simulink model "x_vacc_test"  (Simulink 8.0)
settings: Solver=ode45  StartTime=0.0  StopTime=20*(4096/8)*40  RelTol=1e-3
model workspace (MAT data in the model file):
  ws_data_in.time = [0 1 2 3 4 5 6 7 8 9 10 11 ... (163840 elements, 163840x1)]
  ws_data_in.signals.values = [1 0 0 0 0 0 0 0 0 0 0 0 ... (163840 elements, 163840x1)]
  ws_valid_in.time = [0 1 2 3 4 5 6 7 8 9 10 11 ... (163840 elements, 163840x1)]
  ws_valid_in.signals.values = [1 1 1 1 1 1 1 1 1 1 1 0 ... (163840 elements, 163840x1)]
  ws_dumps_per_acc_in.time = 0
  ws_dumps_per_acc_in.signals.values = 2
libraries: xbsIndex_r4 x531
(part 1 of 4)
settings Model: Creator=davidm  LastModifiedBy=davidm

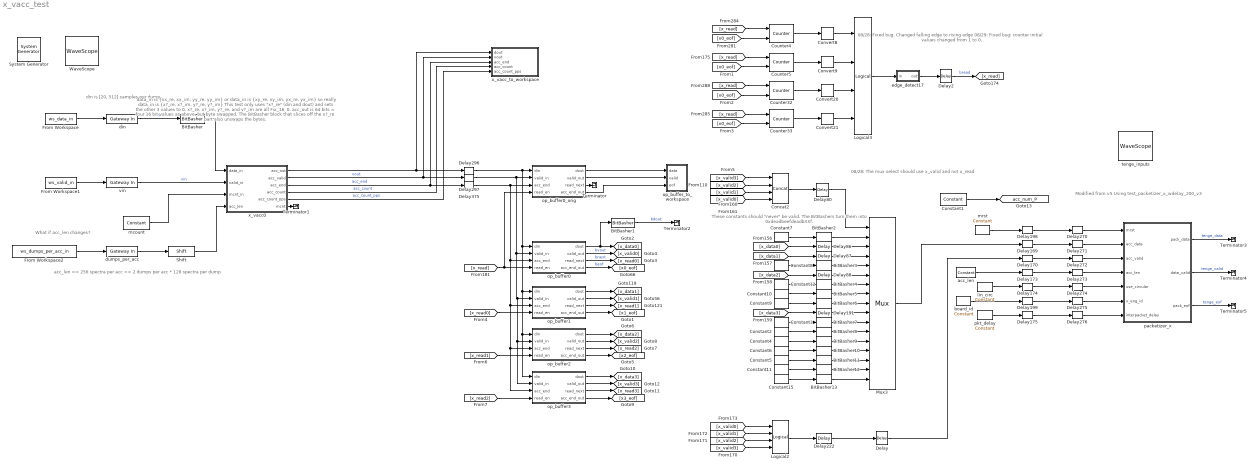
== system x_vacc_test ==
  note: What if acc_len changes?
  note: din is [20, 512] samples per dump
  note: acc_len == 256 spectra per acc == 2 dumps per acc * 128 spectra per dump
  note: data_in  is {xx_re, xx_im, yy_re, yy_im} or
      | data_in  is {xy_re, xy_im, yx_re, yx_im} so really
      | data_in is {x?_re, x?_im, y?_re, y?_im}
      | This test only uses "x?_re" (din and dout) and sets the other 3 values to 0.
      | x?_re, x?_im, y?_re, and y?_im are all Fix_16_0.
      |
      | acc_out is 64 bits = four 16 bit values as above, but byte swapped.
      | The BitBasher block that slices off the x?_re part also unswaps the bytes.
  note: 08/28: Fixed bug. Changed falling edge to rising edge
      | 08/29: Fixed bug: counter initial values changed from 1 to 0.
  note: These constants should "never" be valid.
      | The BitBashers turn them into
      | 0xdeadbeefdeadbXXf.
  note: Modified from v5
      | Using  test_packetizer_x_wdelay_200_v3
  note: 08/28: The mux select should use x_valid and not x_read
  " System Generator" : xbsIndex_r4/ System Generator  Tag=genX  xilinxfamily=Kintex7  part=xc7k325t  speed=-3  package=fbg676  synthesis_tool=XST  clock_wrapper=Clock Enables  directory=./netlist  proj_type=Project Navigator  Synth_file=XST Defaults  Impl_file=ISE Defaults  testbench=off  simulink_period=1  sysclk_period=10  dcm_input_clock_period=10  incr_netlist=off  trim_vbits=Everywhere in SubSystem  dbl_ovrd=According to Block Masks  core_generation=According to Block Masks  run_coregen=off  deprecated_control=off  eval_field=0  block_type=sysgen
  BitBasher : xbsIndex_r4/BitBasher  bitexpr=|  display_expr=off  sr_1=1  arith_type1=Unsigned  bin_pt1=0  sr_2=2  arith_type2=Unsigned  bin_pt2=0  sr_3=3  arith_type3=Unsigned  bin_pt3=0  sr_4=4  arith_type4=Unsigned  bin_pt4=0  dbl_ovrd=off  block_type=bitbasher
      | <!DOCTYPE HTML PUBLIC "-//W3C//DTD HTML 4.0//EN" "http://www.w3.org/TR/REC-html40/strict.dtd">
      | <html><head><meta name="qrichtext" content="1" /><style type="text/css">
      | p, li { white-space: pre-wrap; }
      | </style></head><body style=" font-family:'Sans'; font-size:10pt; font-weight:400; font-style:normal;">
      | <p style=" margin-top:12px; margin-bottom:12px; margin-left:0px; margin-right:0px; -qt-block-indent:0; text-indent:0px;"><span style=" font-family:'MS Shell Dlg'; font-size:8pt;">d64={d, 48'b0}</span></p></body></html>
  BitBasher1 : xbsIndex_r4/BitBasher  bitexpr=|  display_expr=off  sr_1=1  arith_type1="Signed  (2's comp)"  bin_pt1=0  sr_2=2  arith_type2=Unsigned  bin_pt2=0  sr_3=3  arith_type3=Unsigned  bin_pt3=0  sr_4=4  arith_type4=Unsigned  bin_pt4=0  dbl_ovrd=off  block_type=bitbasher
      | <!DOCTYPE HTML PUBLIC "-//W3C//DTD HTML 4.0//EN" "http://www.w3.org/TR/REC-html40/strict.dtd">
      | <html><head><meta name="qrichtext" content="1" /><style type="text/css">
      | p, li { white-space: pre-wrap; }
      | </style></head><body style=" font-family:'Sans'; font-size:10pt; font-weight:400; font-style:normal;">
      | <p style=" margin-top:12px; margin-bottom:12px; margin-left:0px; margin-right:0px; -qt-block-indent:0; text-indent:0px;"><span style=" font-family:'MS Shell Dlg'; font-size:8pt;">d16={d[55:48],d[63:56]}</span></p></body></html>
  BitBasher10 : xbsIndex_r4/BitBasher  bitexpr=|  display_expr=off  sr_1=1  arith_type1=Unsigned  bin_pt1=0  sr_2=2  arith_type2=Unsigned  bin_pt2=0  sr_3=3  arith_type3=Unsigned  bin_pt3=0  sr_4=4  arith_type4=Unsigned  bin_pt4=0  dbl_ovrd=off  block_type=bitbasher
      | <!DOCTYPE HTML PUBLIC "-//W3C//DTD HTML 4.0//EN" "http://www.w3.org/TR/REC-html40/strict.dtd">
      | <html><head><meta name="qrichtext" content="1" /><style type="text/css">
      | p, li { white-space: pre-wrap; }
      | </style></head><body style=" font-family:'Sans'; font-size:10pt; font-weight:400; font-style:normal;">
      | <p style=" margin-top:12px; margin-bottom:12px; margin-left:0px; margin-right:0px; -qt-block-indent:0; text-indent:0px;"><span style=" font-family:'MS Shell Dlg'; font-size:8pt;">o={32'hdeadbeef, 16'hdead, 4'hb, i[3:0], i[3:0], 4'hf}</span></p></body></html>
  BitBasher11 : xbsIndex_r4/BitBasher  bitexpr=|  display_expr=off  sr_1=1  arith_type1=Unsigned  bin_pt1=0  sr_2=2  arith_type2=Unsigned  bin_pt2=0  sr_3=3  arith_type3=Unsigned  bin_pt3=0  sr_4=4  arith_type4=Unsigned  bin_pt4=0  dbl_ovrd=off  block_type=bitbasher
      | <!DOCTYPE HTML PUBLIC "-//W3C//DTD HTML 4.0//EN" "http://www.w3.org/TR/REC-html40/strict.dtd">
      | <html><head><meta name="qrichtext" content="1" /><style type="text/css">
      | p, li { white-space: pre-wrap; }
      | </style></head><body style=" font-family:'Sans'; font-size:10pt; font-weight:400; font-style:normal;">
      | <p style=" margin-top:12px; margin-bottom:12px; margin-left:0px; margin-right:0px; -qt-block-indent:0; text-indent:0px;"><span style=" font-family:'MS Shell Dlg'; font-size:8pt;">o={32'hdeadbeef, 16'hdead, 4'hb, i[3:0], i[3:0], 4'hf}</span></p></body></html>
  BitBasher12 : xbsIndex_r4/BitBasher  bitexpr=|  display_expr=off  sr_1=1  arith_type1=Unsigned  bin_pt1=0  sr_2=2  arith_type2=Unsigned  bin_pt2=0  sr_3=3  arith_type3=Unsigned  bin_pt3=0  sr_4=4  arith_type4=Unsigned  bin_pt4=0  dbl_ovrd=off  block_type=bitbasher
      | <!DOCTYPE HTML PUBLIC "-//W3C//DTD HTML 4.0//EN" "http://www.w3.org/TR/REC-html40/strict.dtd">
      | <html><head><meta name="qrichtext" content="1" /><style type="text/css">
      | p, li { white-space: pre-wrap; }
      | </style></head><body style=" font-family:'Sans'; font-size:10pt; font-weight:400; font-style:normal;">
      | <p style=" margin-top:12px; margin-bottom:12px; margin-left:0px; margin-right:0px; -qt-block-indent:0; text-indent:0px;"><span style=" font-family:'MS Shell Dlg'; font-size:8pt;">o={32'hdeadbeef, 16'hdead, 4'hb, i[3:0], i[3:0], 4'hf}</span></p></body></html>
  BitBasher13 : xbsIndex_r4/BitBasher  bitexpr=|  display_expr=off  sr_1=1  arith_type1=Unsigned  bin_pt1=0  sr_2=2  arith_type2=Unsigned  bin_pt2=0  sr_3=3  arith_type3=Unsigned  bin_pt3=0  sr_4=4  arith_type4=Unsigned  bin_pt4=0  dbl_ovrd=off  block_type=bitbasher
      | <!DOCTYPE HTML PUBLIC "-//W3C//DTD HTML 4.0//EN" "http://www.w3.org/TR/REC-html40/strict.dtd">
      | <html><head><meta name="qrichtext" content="1" /><style type="text/css">
      | p, li { white-space: pre-wrap; }
      | </style></head><body style=" font-family:'Sans'; font-size:10pt; font-weight:400; font-style:normal;">
      | <p style=" margin-top:12px; margin-bottom:12px; margin-left:0px; margin-right:0px; -qt-block-indent:0; text-indent:0px;"><span style=" font-family:'MS Shell Dlg'; font-size:8pt;">o={32'hdeadbeef, 16'hdead, 4'hb, i[3:0], i[3:0], 4'hf}</span></p></body></html>
  BitBasher2 : xbsIndex_r4/BitBasher  bitexpr=|  display_expr=off  sr_1=1  arith_type1=Unsigned  bin_pt1=0  sr_2=2  arith_type2=Unsigned  bin_pt2=0  sr_3=3  arith_type3=Unsigned  bin_pt3=0  sr_4=4  arith_type4=Unsigned  bin_pt4=0  dbl_ovrd=off  block_type=bitbasher
      | <!DOCTYPE HTML PUBLIC "-//W3C//DTD HTML 4.0//EN" "http://www.w3.org/TR/REC-html40/strict.dtd">
      | <html><head><meta name="qrichtext" content="1" /><style type="text/css">
      | p, li { white-space: pre-wrap; }
      | </style></head><body style=" font-family:'Sans'; font-size:10pt; font-weight:400; font-style:normal;">
      | <p style=" margin-top:12px; margin-bottom:12px; margin-left:0px; margin-right:0px; -qt-block-indent:0; text-indent:0px;"><span style=" font-family:'MS Shell Dlg'; font-size:8pt;">o={32'hdeadbeef, 16'hdead, 4'hb, i[3:0], i[3:0], 4'hf}</span></p></body></html>
  BitBasher3 : xbsIndex_r4/BitBasher  bitexpr=|  display_expr=off  sr_1=1  arith_type1=Unsigned  bin_pt1=0  sr_2=2  arith_type2=Unsigned  bin_pt2=0  sr_3=3  arith_type3=Unsigned  bin_pt3=0  sr_4=4  arith_type4=Unsigned  bin_pt4=0  dbl_ovrd=off  block_type=bitbasher
      | <!DOCTYPE HTML PUBLIC "-//W3C//DTD HTML 4.0//EN" "http://www.w3.org/TR/REC-html40/strict.dtd">
      | <html><head><meta name="qrichtext" content="1" /><style type="text/css">
      | p, li { white-space: pre-wrap; }
      | </style></head><body style=" font-family:'Sans'; font-size:10pt; font-weight:400; font-style:normal;">
      | <p style=" margin-top:12px; margin-bottom:12px; margin-left:0px; margin-right:0px; -qt-block-indent:0; text-indent:0px;"><span style=" font-family:'MS Shell Dlg'; font-size:8pt;">o={32'hdeadbeef, 16'hdead, 4'hb, i[3:0], i[3:0], 4'hf}</span></p></body></html>
  BitBasher4 : xbsIndex_r4/BitBasher  bitexpr=|  display_expr=off  sr_1=1  arith_type1=Unsigned  bin_pt1=0  sr_2=2  arith_type2=Unsigned  bin_pt2=0  sr_3=3  arith_type3=Unsigned  bin_pt3=0  sr_4=4  arith_type4=Unsigned  bin_pt4=0  dbl_ovrd=off  block_type=bitbasher
      | <!DOCTYPE HTML PUBLIC "-//W3C//DTD HTML 4.0//EN" "http://www.w3.org/TR/REC-html40/strict.dtd">
      | <html><head><meta name="qrichtext" content="1" /><style type="text/css">
      | p, li { white-space: pre-wrap; }
      | </style></head><body style=" font-family:'Sans'; font-size:10pt; font-weight:400; font-style:normal;">
      | <p style=" margin-top:12px; margin-bottom:12px; margin-left:0px; margin-right:0px; -qt-block-indent:0; text-indent:0px;"><span style=" font-family:'MS Shell Dlg'; font-size:8pt;">o={32'hdeadbeef, 16'hdead, 4'hb, i[3:0], i[3:0], 4'hf}</span></p></body></html>
  BitBasher5 : xbsIndex_r4/BitBasher  bitexpr=|  display_expr=off  sr_1=1  arith_type1=Unsigned  bin_pt1=0  sr_2=2  arith_type2=Unsigned  bin_pt2=0  sr_3=3  arith_type3=Unsigned  bin_pt3=0  sr_4=4  arith_type4=Unsigned  bin_pt4=0  dbl_ovrd=off  block_type=bitbasher
      | <!DOCTYPE HTML PUBLIC "-//W3C//DTD HTML 4.0//EN" "http://www.w3.org/TR/REC-html40/strict.dtd">
      | <html><head><meta name="qrichtext" content="1" /><style type="text/css">
      | p, li { white-space: pre-wrap; }
      | </style></head><body style=" font-family:'Sans'; font-size:10pt; font-weight:400; font-style:normal;">
      | <p style=" margin-top:12px; margin-bottom:12px; margin-left:0px; margin-right:0px; -qt-block-indent:0; text-indent:0px;"><span style=" font-family:'MS Shell Dlg'; font-size:8pt;">o={32'hdeadbeef, 16'hdead, 4'hb, i[3:0], i[3:0], 4'hf}</span></p></body></html>
  BitBasher6 : xbsIndex_r4/BitBasher  bitexpr=|  display_expr=off  sr_1=1  arith_type1=Unsigned  bin_pt1=0  sr_2=2  arith_type2=Unsigned  bin_pt2=0  sr_3=3  arith_type3=Unsigned  bin_pt3=0  sr_4=4  arith_type4=Unsigned  bin_pt4=0  dbl_ovrd=off  block_type=bitbasher
      | <!DOCTYPE HTML PUBLIC "-//W3C//DTD HTML 4.0//EN" "http://www.w3.org/TR/REC-html40/strict.dtd">
      | <html><head><meta name="qrichtext" content="1" /><style type="text/css">
      | p, li { white-space: pre-wrap; }
      | </style></head><body style=" font-family:'Sans'; font-size:10pt; font-weight:400; font-style:normal;">
      | <p style=" margin-top:12px; margin-bottom:12px; margin-left:0px; margin-right:0px; -qt-block-indent:0; text-indent:0px;"><span style=" font-family:'MS Shell Dlg'; font-size:8pt;">o={32'hdeadbeef, 16'hdead, 4'hb, i[3:0], i[3:0], 4'hf}</span></p></body></html>
  BitBasher7 : xbsIndex_r4/BitBasher  bitexpr=|  display_expr=off  sr_1=1  arith_type1=Unsigned  bin_pt1=0  sr_2=2  arith_type2=Unsigned  bin_pt2=0  sr_3=3  arith_type3=Unsigned  bin_pt3=0  sr_4=4  arith_type4=Unsigned  bin_pt4=0  dbl_ovrd=off  block_type=bitbasher
      | <!DOCTYPE HTML PUBLIC "-//W3C//DTD HTML 4.0//EN" "http://www.w3.org/TR/REC-html40/strict.dtd">
      | <html><head><meta name="qrichtext" content="1" /><style type="text/css">
      | p, li { white-space: pre-wrap; }
      | </style></head><body style=" font-family:'Sans'; font-size:10pt; font-weight:400; font-style:normal;">
      | <p style=" margin-top:12px; margin-bottom:12px; margin-left:0px; margin-right:0px; -qt-block-indent:0; text-indent:0px;"><span style=" font-family:'MS Shell Dlg'; font-size:8pt;">o={32'hdeadbeef, 16'hdead, 4'hb, i[3:0], i[3:0], 4'hf}</span></p></body></html>
  BitBasher8 : xbsIndex_r4/BitBasher  bitexpr=|  display_expr=off  sr_1=1  arith_type1=Unsigned  bin_pt1=0  sr_2=2  arith_type2=Unsigned  bin_pt2=0  sr_3=3  arith_type3=Unsigned  bin_pt3=0  sr_4=4  arith_type4=Unsigned  bin_pt4=0  dbl_ovrd=off  block_type=bitbasher
      | <!DOCTYPE HTML PUBLIC "-//W3C//DTD HTML 4.0//EN" "http://www.w3.org/TR/REC-html40/strict.dtd">
      | <html><head><meta name="qrichtext" content="1" /><style type="text/css">
      | p, li { white-space: pre-wrap; }
      | </style></head><body style=" font-family:'Sans'; font-size:10pt; font-weight:400; font-style:normal;">
      | <p style=" margin-top:12px; margin-bottom:12px; margin-left:0px; margin-right:0px; -qt-block-indent:0; text-indent:0px;"><span style=" font-family:'MS Shell Dlg'; font-size:8pt;">o={32'hdeadbeef, 16'hdead, 4'hb, i[3:0], i[3:0], 4'hf}</span></p></body></html>
  BitBasher9 : xbsIndex_r4/BitBasher  bitexpr=|  display_expr=off  sr_1=1  arith_type1=Unsigned  bin_pt1=0  sr_2=2  arith_type2=Unsigned  bin_pt2=0  sr_3=3  arith_type3=Unsigned  bin_pt3=0  sr_4=4  arith_type4=Unsigned  bin_pt4=0  dbl_ovrd=off  block_type=bitbasher
      | <!DOCTYPE HTML PUBLIC "-//W3C//DTD HTML 4.0//EN" "http://www.w3.org/TR/REC-html40/strict.dtd">
      | <html><head><meta name="qrichtext" content="1" /><style type="text/css">
      | p, li { white-space: pre-wrap; }
      | </style></head><body style=" font-family:'Sans'; font-size:10pt; font-weight:400; font-style:normal;">
      | <p style=" margin-top:12px; margin-bottom:12px; margin-left:0px; margin-right:0px; -qt-block-indent:0; text-indent:0px;"><span style=" font-family:'MS Shell Dlg'; font-size:8pt;">o={32'hdeadbeef, 16'hdead, 4'hb, i[3:0], i[3:0], 4'hf}</span></p></body></html>
  Concat2 : xbsIndex_r4/Concat  num_inputs=4  dbl_ovrd=off  block_type=concat
      settings: block_version=10.1.3
  Constant1 : xbsIndex_r4/Constant  const=0  gui_display_data_type=Fixed-point  arith_type=Unsigned  n_bits=32  bin_pt=0  preci_type=Single  exp_width=8  frac_width=24  explicit_period=on  period=1  equ=P=C  opselect=C  inp2=PCIN>>17  opr=+  inp1=P  carry=CIN  dbl_ovrd=off  block_type=constant
      settings: block_version=11.4
  Constant10 : xbsIndex_r4/Constant  const=6  gui_display_data_type=Fixed-point  arith_type=Unsigned  n_bits=4  bin_pt=0  preci_type=Single  exp_width=8  frac_width=24  explicit_period=on  period=1  equ=P=C  opselect=C  inp2=PCIN>>17  opr=+  inp1=P  carry=CIN  dbl_ovrd=off  block_type=constant
      settings: block_version=11.4
  Constant11 : xbsIndex_r4/Constant  const=14  gui_display_data_type=Fixed-point  arith_type=Unsigned  n_bits=4  bin_pt=0  preci_type=Single  exp_width=8  frac_width=24  explicit_period=on  period=1  equ=P=C  opselect=C  inp2=PCIN>>17  opr=+  inp1=P  carry=CIN  dbl_ovrd=off  block_type=constant
      settings: block_version=11.4
  Constant12 : xbsIndex_r4/Constant  const=5  gui_display_data_type=Fixed-point  arith_type=Unsigned  n_bits=4  bin_pt=0  preci_type=Single  exp_width=8  frac_width=24  explicit_period=on  period=1  equ=P=C  opselect=C  inp2=PCIN>>17  opr=+  inp1=P  carry=CIN  dbl_ovrd=off  block_type=constant
      settings: block_version=11.4
  Constant15 : xbsIndex_r4/Constant  const=15  gui_display_data_type=Fixed-point  arith_type=Unsigned  n_bits=4  bin_pt=0  preci_type=Single  exp_width=8  frac_width=24  explicit_period=on  period=1  equ=P=C  opselect=C  inp2=PCIN>>17  opr=+  inp1=P  carry=CIN  dbl_ovrd=off  block_type=constant
      settings: block_version=11.4
  Constant2 : xbsIndex_r4/Constant  const=10  gui_display_data_type=Fixed-point  arith_type=Unsigned  n_bits=4  bin_pt=0  preci_type=Single  exp_width=8  frac_width=24  explicit_period=on  period=1  equ=P=C  opselect=C  inp2=PCIN>>17  opr=+  inp1=P  carry=CIN  dbl_ovrd=off  block_type=constant
      settings: block_version=11.4
  Constant3 : xbsIndex_r4/Constant  const=9  gui_display_data_type=Fixed-point  arith_type=Unsigned  n_bits=4  bin_pt=0  preci_type=Single  exp_width=8  frac_width=24  explicit_period=on  period=1  equ=P=C  opselect=C  inp2=PCIN>>17  opr=+  inp1=P  carry=CIN  dbl_ovrd=off  block_type=constant
      settings: block_version=11.4
  Constant4 : xbsIndex_r4/Constant  const=11  gui_display_data_type=Fixed-point  arith_type=Unsigned  n_bits=4  bin_pt=0  preci_type=Single  exp_width=8  frac_width=24  explicit_period=on  period=1  equ=P=C  opselect=C  inp2=PCIN>>17  opr=+  inp1=P  carry=CIN  dbl_ovrd=off  block_type=constant
      settings: block_version=11.4
  Constant5 : xbsIndex_r4/Constant  const=13  gui_display_data_type=Fixed-point  arith_type=Unsigned  n_bits=4  bin_pt=0  preci_type=Single  exp_width=8  frac_width=24  explicit_period=on  period=1  equ=P=C  opselect=C  inp2=PCIN>>17  opr=+  inp1=P  carry=CIN  dbl_ovrd=off  block_type=constant
      settings: block_version=11.4
  Constant6 : xbsIndex_r4/Constant  const=12  gui_display_data_type=Fixed-point  arith_type=Unsigned  n_bits=4  bin_pt=0  preci_type=Single  exp_width=8  frac_width=24  explicit_period=on  period=1  equ=P=C  opselect=C  inp2=PCIN>>17  opr=+  inp1=P  carry=CIN  dbl_ovrd=off  block_type=constant
      settings: block_version=11.4
  Constant7 : xbsIndex_r4/Constant  const=0  gui_display_data_type=Fixed-point  arith_type=Unsigned  n_bits=4  bin_pt=0  preci_type=Single  exp_width=8  frac_width=24  explicit_period=on  period=1  equ=P=C  opselect=C  inp2=PCIN>>17  opr=+  inp1=P  carry=CIN  dbl_ovrd=off  block_type=constant
      settings: block_version=11.4
  Constant8 : xbsIndex_r4/Constant  const=3  gui_display_data_type=Fixed-point  arith_type=Unsigned  n_bits=4  bin_pt=0  preci_type=Single  exp_width=8  frac_width=24  explicit_period=on  period=1  equ=P=C  opselect=C  inp2=PCIN>>17  opr=+  inp1=P  carry=CIN  dbl_ovrd=off  block_type=constant
      settings: block_version=11.4
  Constant9 : xbsIndex_r4/Constant  const=7  gui_display_data_type=Fixed-point  arith_type=Unsigned  n_bits=4  bin_pt=0  preci_type=Single  exp_width=8  frac_width=24  explicit_period=on  period=1  equ=P=C  opselect=C  inp2=PCIN>>17  opr=+  inp1=P  carry=CIN  dbl_ovrd=off  block_type=constant
      settings: block_version=11.4
  Convert{8..9,20..21} (4 alike) : xbsIndex_r4/Convert  gui_display_data_type=Fixed-point  arith_type=Boolean  n_bits=16  bin_pt=14  float_type=Single  exp_bits=8  fraction_bits=24  quantization=Truncate  overflow=Wrap  en=off  latency=0  dbl_ovrd=off  pipeline=on  block_type=convert
      settings: block_version=11.4
  Counter{4..5,32..33} (4 alike) : xbsIndex_r4/Counter  cnt_type=Free Running  cnt_to=Inf  operation=Up  start_count=0  cnt_by_val=1  arith_type=Unsigned  n_bits=1  bin_pt=0  load_pin=off  rst=on  en=on  explicit_period=off  period=1  dbl_ovrd=off  use_behavioral_HDL=off  implementation=Fabric  block_type=counter
      settings: block_version=11.4
  Delay{,2,80,86..88,191,222,270..276,296..297,375} (18 alike) : xbsIndex_r4/Delay  rst=off  infoeditControl=Selection of Reset will increase slice count due to use of real FFs and instead of SRLs  en=off  latency=1  dbl_ovrd=off  reg_retiming=on  block_type=delay
      settings: block_version=11.4
  Delay{169..170,173..175,198..199} (7 alike) : xbsIndex_r4/Delay  rst=off  infoeditControl=Selection of Reset will increase slice count due to use of real FFs and instead of SRLs  en=off  latency=2  dbl_ovrd=off  reg_retiming=on  block_type=delay
      settings: block_version=11.4
  From Workspace : FromWorkspace  VariableName=ws_data_in  SampleTime=1  Interpolate=off  OutputAfterFinalValue=Holding final value
  From Workspace1 : FromWorkspace  VariableName=ws_valid_in  SampleTime=1  Interpolate=off  OutputAfterFinalValue=Holding final value
  From Workspace2 : FromWorkspace  VariableName=ws_dumps_per_acc_in  SampleTime=1  Interpolate=off  OutputAfterFinalValue=Holding final value
  Logical2 : xbsIndex_r4/Logical  logical_function=OR  inputs=4  en=off  latency=0  precision=Full  arith_type=Unsigned  n_bits=16  bin_pt=0  align_bp=on  dbl_ovrd=off  block_type=logical
      settings: block_version=11.4
  Logical3 : xbsIndex_r4/Logical  logical_function=AND  inputs=4  en=off  latency=0  precision=Full  arith_type=Unsigned  n_bits=16  bin_pt=0  align_bp=on  dbl_ovrd=off  block_type=logical
      settings: block_version=11.4
  Mux3 : xbsIndex_r4/Mux  inputs=16  en=off  latency=1  precision=Full  arith_type=Unsigned  n_bits=16  bin_pt=14  quantization=Truncate  overflow=Wrap  dbl_ovrd=off  block_type=mux
      settings: block_version=11.4
  Shift : xbsIndex_r4/Shift  shift_dir=Left  shift_bits=7  en=off  latency=0  precision=Full  arith_type="Signed  (2's comp)"  n_bits=16  bin_pt=14  quantization=Truncate  overflow=Wrap  dbl_ovrd=off  block_type=shift
  Terminator{,1..5} (6 alike) : Terminator
  WaveScope : xbsIndex_r4/WaveScope  block_type=wavescope
  acc_len : xbsIndex_r4/Constant  const=2  gui_display_data_type=Fixed-point  arith_type=Unsigned  n_bits=16  bin_pt=0  preci_type=Single  exp_width=8  frac_width=24  explicit_period=on  period=1  equ=P=C  opselect=C  inp2=PCIN>>17  opr=+  inp1=P  carry=CIN  dbl_ovrd=off  block_type=constant
      settings: block_version=11.4
  board_id : xbsIndex_r4/Constant  const=0  gui_display_data_type=Fixed-point  arith_type=Unsigned  n_bits=4  bin_pt=0  preci_type=Single  exp_width=8  frac_width=24  explicit_period=on  period=1  equ=P=C  opselect=C  inp2=PCIN>>17  opr=+  inp1=P  carry=CIN  dbl_ovrd=off  block_type=constant
      settings: block_version=11.4
  din : xbsIndex_r4/Gateway In  gui_display_data_type=Fixed-point  arith_type="Signed  (2's comp)"  n_bits=16  bin_pt=0  preci_type=Single  exp_width=8  frac_width=24  quantization="Round  (unbiased: +/- Inf)"  overflow=Saturate  period=1  dbl_ovrd=off  timing_constraint=None  locs_specified=off  LOCs={}  UseAsADC=off  ADCChannel='1'  inherit_from_input=off  hdl_port=on  block_type=gatewayin
  dumps_per_acc : xbsIndex_r4/Gateway In  gui_display_data_type=Fixed-point  arith_type=Unsigned  n_bits=16  bin_pt=0  preci_type=Single  exp_width=8  frac_width=24  quantization="Round  (unbiased: +/- Inf)"  overflow=Saturate  period=1  dbl_ovrd=off  timing_constraint=None  locs_specified=off  LOCs={}  UseAsADC=off  ADCChannel='1'  inherit_from_input=off  hdl_port=on  block_type=gatewayin
  edge_detect17 : Subsystem  AncestorBlock=casper_library_misc/edge_detect  mask(edge_detect){edge=Rising, polarity=Active High, x_in=[0 0.08 0.08 0.16 0.16 0.24 0.24 0.32 0.32 0.4], y_in=[-1 -1  1  1  1  1  1  1  1  1], x_out=[0.6 0.68 0.68 0.76 0.76 0.84 0.84 0.92 0.92 1], y_out=[-1 -1  1  1 -1 -1 -1 -1 -1 -1]}
      mask prompts: edge "Edge Type"; polarity "Output Polarity"; x_in "Input X Positions"; y_in "Input Y Positions"; x_out "Output X Positions"; y_out "Output Y Positions"
      mask help: Output is active for one-cycle after each edge of the specified type (i.e. rising, falling or both).
      mask init:
      | edge_detect_init(gcb,...
      |     'edge',edge,...
      |     'polarity',polarity,...
      |     'x_in',x_in,...
      |     'x_out',x_out,...
      |     'y_in',y_in,...
      |     'y_out',y_out);
      | 
      | x_in_num=str2num(getfield(getfield(get_param(gcb,'UserData'),'parameters'),'x_in'));
      | x_out_num=str2num(getfield(getfield(get_param(gcb,'UserData'),'parameters'),'x_out'));
      | y_in_num=str2num(getfield(getfield(get_param(gcb,'UserData'),'parameters'),'y_in'));
      | y_out_num=str2num(getfield(getfield(get_param(gcb,'UserData'),'parameters'),'y_out'));
      settings: AttributesFormatString=|
      | rising edges
      | active high
      mask Help: Output is active for one-cycle after each edge of the specified type (i.e. rising, falling, or both).  Latency is zero.
      mask TypeOptions edge: Rising | Falling | Both
      mask TypeOptions polarity: Active High | Active Low
      mask Display:
      | plot(x_in_num,0.5+y_in_num*0.2);
      | plot(x_out_num,0.5+y_out_num*0.2);
  lin_circ : xbsIndex_r4/Constant  const=0  gui_display_data_type=Fixed-point  arith_type=Unsigned  n_bits=1  bin_pt=0  preci_type=Single  exp_width=8  frac_width=24  explicit_period=on  period=1  equ=P=C  opselect=C  inp2=PCIN>>17  opr=+  inp1=P  carry=CIN  dbl_ovrd=off  block_type=constant
      settings: block_version=11.4
  mcount : xbsIndex_r4/Constant  const=1  gui_display_data_type=Fixed-point  arith_type=Unsigned  n_bits=48  bin_pt=0  preci_type=Single  exp_width=8  frac_width=24  explicit_period=on  period=1  equ=P=C  opselect=C  inp2=PCIN>>17  opr=+  inp1=P  carry=CIN  dbl_ovrd=off  block_type=constant
  mrst : xbsIndex_r4/Constant  const=0  gui_display_data_type=Boolean  arith_type=Boolean  n_bits=4  bin_pt=0  preci_type=Single  exp_width=8  frac_width=24  explicit_period=on  period=1  equ=P=C  opselect=C  inp2=PCIN>>17  opr=+  inp1=P  carry=CIN  dbl_ovrd=off  block_type=constant
      settings: block_version=11.4
  op_buffer0 : Subsystem  mask{freq_chan=12, n_x_eng=3, n_x_op=22, n_buffer=1, bram_latency=2}
      mask prompts: freq_chan "No. of Frequency Channels in the system (2 ^ ?)"; n_x_eng "No. of X-engines in the system (2 ^ ?)"; n_x_op "No. of X-engine outputs"; n_buffer "No. of accumulations to buffer"; bram_latency "BRAM latency"
      mask init:
      | req_depth = (n_x_op/2) * (2 ^ (freq_chan - n_x_eng));
      | fifo_depth = 2^nextpow2(req_depth);
      | if fifo_depth < 1024
      |   set_param([gcb,'/FIFO'], 'depth', sprintf('%d', fifo_depth));
      | else
      |   set_param([gcb,'/FIFO'], 'depth', sprintf('%dK', fifo_depth/1024));
      | end
  op_buffer0_orig : Subsystem  mask{freq_chan=12, n_x_eng=3, n_x_op=22, n_buffer=1, bram_latency=2}
      mask prompts: freq_chan "No. of Frequency Channels in the system (2 ^ ?)"; n_x_eng "No. of X-engines in the system (2 ^ ?)"; n_x_op "No. of X-engine outputs"; n_buffer "No. of accumulations to buffer"; bram_latency "BRAM latency"
  op_buffer1 : Subsystem  mask{freq_chan=12, n_x_eng=3, n_x_op=22, n_buffer=1, bram_latency=2}
      mask prompts: freq_chan "No. of Frequency Channels in the system (2 ^ ?)"; n_x_eng "No. of X-engines in the system (2 ^ ?)"; n_x_op "No. of X-engine outputs"; n_buffer "No. of accumulations to buffer"; bram_latency "BRAM latency"
      mask init: same as x_vacc_test/op_buffer0
  op_buffer2 : Subsystem  mask{freq_chan=12, n_x_eng=3, n_x_op=22, n_buffer=1, bram_latency=2}
      mask prompts: freq_chan "No. of Frequency Channels in the system (2 ^ ?)"; n_x_eng "No. of X-engines in the system (2 ^ ?)"; n_x_op "No. of X-engine outputs"; n_buffer "No. of accumulations to buffer"; bram_latency "BRAM latency"
      mask init: same as x_vacc_test/op_buffer0
  op_buffer3 : Subsystem  mask{freq_chan=12, n_x_eng=3, n_x_op=22, n_buffer=1, bram_latency=2}
      mask prompts: freq_chan "No. of Frequency Channels in the system (2 ^ ?)"; n_x_eng "No. of X-engines in the system (2 ^ ?)"; n_x_op "No. of X-engine outputs"; n_buffer "No. of accumulations to buffer"; bram_latency "BRAM latency"
      mask init: same as x_vacc_test/op_buffer0
  op_buffer_to_workspace : Subsystem
  packetizer_x : Subsystem  mask{header_len=88, n_packets=128, freq_chan=12, n_x_op=22, n_x=1}
      mask prompts: header_len "Packet header length (bytes)"; n_packets "No. of packets per accumulation"; freq_chan "Total frequency channels ( 2 ^ ? )"; n_x_op "Number of X-engine Output"; n_x "Number of ROACH boards (2 ^ ?)"
      mask init:
      | n_freq_chan = 2 ^ (freq_chan - n_x);
      | data_len = n_x_op * n_freq_chan * 4 / n_packets;
  pkt_delay : xbsIndex_r4/Constant  const=100  gui_display_data_type=Fixed-point  arith_type=Unsigned  n_bits=8  bin_pt=0  preci_type=Single  exp_width=8  frac_width=24  explicit_period=on  period=1  equ=P=C  opselect=C  inp2=PCIN>>17  opr=+  inp1=P  carry=CIN  dbl_ovrd=off  block_type=constant
      settings: block_version=11.4
  tenge_inputs : xbsIndex_r4/WaveScope  block_type=wavescope
  vin : xbsIndex_r4/Gateway In  gui_display_data_type=Boolean  arith_type=Boolean  n_bits=16  bin_pt=14  preci_type=Single  exp_width=8  frac_width=24  quantization="Round  (unbiased: +/- Inf)"  overflow=Saturate  period=1  dbl_ovrd=off  timing_constraint=None  locs_specified=off  LOCs={}  UseAsADC=off  ADCChannel='1'  inherit_from_input=off  hdl_port=on  block_type=gatewayin
  x_vacc0 : Subsystem  mask{x_acc_bits=7, x_bin_pt=6, freq_chan=12, n_x_eng=3, x_n_op=22, bram_latency=3}
      mask prompts: x_acc_bits "X-engine Accumulation (2 ^ ?)"; x_bin_pt "X-engine Output Binary point"; freq_chan "Frequency channels in the system (2 ^ ?)"; n_x_eng "Total no. of X-engines in the system (2 ^ ?)"; x_n_op "No. of X outputs"; bram_latency "BRAM Latency"
      mask init:
      | x_freq_chan = freq_chan - n_x_eng;
      | x_len = x_n_op / 2 * (2 ^ x_freq_chan);
  x_vacc_to_workspace : Subsystem
  tag scope: acc_num_P=global
  wires:
  mcount/1 -> x_vacc0/3(mcnt_in)
  Shift/1 -> x_vacc0/4(acc_len)
  vin/1 -> x_vacc0/2(valid_in)  "vin"
  din/1 -> BitBasher/1  "din"
  BitBasher/1 -> x_vacc0/1(data_in)
  From Workspace/1 -> din/1
  From Workspace1/1 -> vin/1
  From Workspace2/1 -> dumps_per_acc/1
  dumps_per_acc/1 -> Shift/1
  x_vacc0/2(acc_valid) -> Delay297/1, x_vacc_to_workspace/2(vout)  "vout"
  x_vacc0/3(acc_end) -> Delay375/1, x_vacc_to_workspace/3(acc_end)  "acc_end"
  x_vacc0/4(acc_count) -> x_vacc_to_workspace/4(acc_count)  "acc_count"
  x_vacc0/5(acc_count_pps) -> x_vacc_to_workspace/5(acc_count_pps)  "acc_count_pps"
  op_buffer0_orig/2(valid_out) -> op_buffer_to_workspace/2(valid)
  op_buffer0_orig/1(dout) -> op_buffer_to_workspace/1(data)
  x_vacc0/1(acc_out) -> Delay296/1, x_vacc_to_workspace/1(dout)
  [x_read] -> op_buffer0_orig/4(read_en), op_buffer0/4(read_en)
  Delay296/1 -> op_buffer0_orig/1(din), op_buffer3/1(din), op_buffer2/1(din), op_buffer1/1(din), op_buffer0/1(din)
  Delay375/1 -> op_buffer0_orig/3(acc_end), op_buffer3/3(acc_end), op_buffer2/3(acc_end), op_buffer1/3(acc_end), op_buffer0/3(acc_end)
  op_buffer0_orig/4(acc_end_out) -> op_buffer_to_workspace/3(eof)
  edge_detect17/1(out) -> Delay2/1
  [x0_eof] -> Counter4/2
  [x0_eof] -> Counter5/2
  Counter4/1 -> Convert8/1
  Counter5/1 -> Convert9/1
  Logical3/1 -> edge_detect17/1(in)
  [x_read] -> Counter5/1
  [x_read] -> Counter4/1
  Delay2/1 -> [x_read]  "bread"
  Convert8/1 -> Logical3/1
  Convert9/1 -> Logical3/2
  [x0_eof] -> Counter32/2
  Counter32/1 -> Convert20/1
  Counter33/1 -> Convert21/1
  [x_read] -> Counter33/1
  [x_read] -> Counter32/1
  Convert20/1 -> Logical3/3
  Convert21/1 -> Logical3/4
  [x0_eof] -> Counter33/2
  op_buffer0_orig/3(read_next) -> Terminator/1
  x_vacc0/6(mcnt) -> Terminator1/1
  op_buffer0/1(dout) -> [x_data0], BitBasher1/1
  op_buffer0/2(valid_out) -> [x_valid0]  "bvout"
  op_buffer0/3(read_next) -> [x_read0]  "bnext"
  op_buffer0/4(acc_end_out) -> [x0_eof]  "beof"
  BitBasher1/1 -> Terminator2/1  "bdout"
  acc_len/1 -> Delay173/1
  lin_circ/1 -> Delay174/1
  board_id/1 -> Delay199/1
  mrst/1 -> Delay198/1
  Delay198/1 -> Delay270/1
  Delay169/1 -> Delay271/1
  Delay170/1 -> Delay272/1
  Delay173/1 -> Delay273/1
  Delay174/1 -> Delay274/1
  Delay199/1 -> Delay275/1
  pkt_delay/1 -> Delay175/1
  Delay175/1 -> Delay276/1
  Delay/1 -> Delay170/1
  Logical2/1 -> Delay222/1
  [x_data0] -> Delay86/1
  [x_valid0] -> Logical2/1
  [x_valid1] -> Logical2/2
  [x_valid2] -> Logical2/3
  [x_valid3] -> Logical2/4
  Mux3/1 -> Delay169/1
  Delay270/1 -> packetizer_x/1(mrst)
  Delay271/1 -> packetizer_x/2(acc_data)
  Delay272/1 -> packetizer_x/3(acc_valid)
  Delay273/1 -> packetizer_x/4(acc_len)
  Delay274/1 -> packetizer_x/5(use_circular)
  Delay275/1 -> packetizer_x/6(x_eng_id)
  Delay276/1 -> packetizer_x/7(interpacket_delay)
  Constant7/1 -> BitBasher2/1
  Delay86/1 -> Mux3/3
  [x_data1] -> Delay87/1
  [x_data2] -> Delay88/1
  [x_data3] -> Delay191/1
  Delay87/1 -> Mux3/4
  Constant8/1 -> BitBasher3/1
  Delay88/1 -> Mux3/6
  Delay191/1 -> Mux3/10
  Delay80/1 -> Mux3/1
  Delay222/1 -> Delay/1
  Concat2/1 -> Delay80/1
  [x_valid3] -> Concat2/1
  [x_valid2] -> Concat2/2
  [x_valid1] -> Concat2/3
  [x_valid0] -> Concat2/4
  BitBasher2/1 -> Mux3/2
  BitBasher3/1 -> Mux3/5
  Constant12/1 -> BitBasher4/1
  Constant10/1 -> BitBasher5/1
  Constant9/1 -> BitBasher6/1
  [x_read0] -> op_buffer1/4(read_en)
  op_buffer1/4(acc_end_out) -> [x1_eof]
  op_buffer1/1(dout) -> [x_data1]
  op_buffer1/2(valid_out) -> [x_valid1]
  op_buffer1/3(read_next) -> [x_read1]
  [x_read1] -> op_buffer2/4(read_en)
  op_buffer2/4(acc_end_out) -> [x2_eof]
  op_buffer2/1(dout) -> [x_data2]
  op_buffer2/2(valid_out) -> [x_valid2]
  op_buffer2/3(read_next) -> [x_read2]
  [x_read2] -> op_buffer3/4(read_en)
  op_buffer3/4(acc_end_out) -> [x3_eof]
  op_buffer3/1(dout) -> [x_data3]
  op_buffer3/2(valid_out) -> [x_valid3]
  op_buffer3/3(read_next) -> [x_read3]
  Delay297/1 -> op_buffer3/2(valid_in), op_buffer2/2(valid_in), op_buffer1/2(valid_in), op_buffer0/2(valid_in), op_buffer0_orig/2(valid_in)
  packetizer_x/1(pack_data) -> Terminator3/1  "tenge_data"
  packetizer_x/2(data_valid) -> Terminator4/1  "tenge_valid"
  packetizer_x/3(pack_eof) -> Terminator5/1  "tenge_eof"
  Constant1/1 -> [acc_num_P]
  BitBasher4/1 -> Mux3/7
  BitBasher5/1 -> Mux3/8
  BitBasher6/1 -> Mux3/9
  Constant3/1 -> BitBasher7/1
  Constant2/1 -> BitBasher8/1
  Constant4/1 -> BitBasher9/1
  BitBasher7/1 -> Mux3/11
  BitBasher8/1 -> Mux3/12
  BitBasher9/1 -> Mux3/13
  Constant6/1 -> BitBasher10/1
  Constant5/1 -> BitBasher11/1
  Constant11/1 -> BitBasher12/1
  BitBasher10/1 -> Mux3/14
  BitBasher11/1 -> Mux3/15
  BitBasher12/1 -> Mux3/16
  Constant15/1 -> BitBasher13/1
  BitBasher13/1 -> Mux3/17

== system x_vacc_test/edge_detect17 ==
  in : Inport
  Delay : xbsIndex_r4/Delay  rst=off  infoeditControl=Selection of Reset will increase slice count due to use of real FFs and instead of SRLs  en=off  latency=1  dbl_ovrd=off  reg_retiming=on  block_type=delay
      settings: xl_area=[0, 0, 0, 0, 0, 0, 0]
  Inverter : xbsIndex_r4/Inverter  en=off  latency=0  dbl_ovrd=off  block_type=inv
      settings: xl_area=[0, 0, 0, 0, 0, 0, 0]
  edge_op : xbsIndex_r4/Logical  logical_function=NOR  inputs=2  en=off  latency=0  precision=Full  arith_type=Unsigned  n_bits=8  bin_pt=2  align_bp=on  dbl_ovrd=off  block_type=logical
      settings: xl_area=[0, 0, 0, 0, 0, 0, 0]
  out : Outport
  wires:
  Inverter/1 -> edge_op/1
  edge_op/1 -> out/1
  in/1 -> Inverter/1, Delay/1
  Delay/1 -> edge_op/2

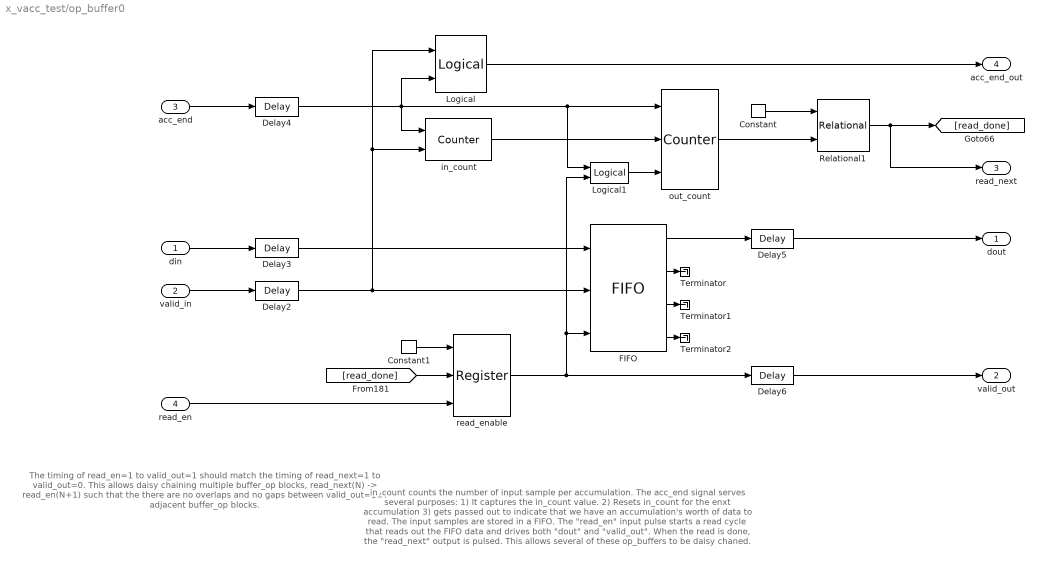
== system x_vacc_test/op_buffer0 ==
  note: The timing of read_en=1 to valid_out=1 should
      | match the timing of read_next=1 to valid_out=0.
      |
      | This allows daisy chaining multiple buffer_op blocks,
      | read_next(N) -> read_en(N+1) such that the there are
      | no overlaps and no gaps between valid_out=1 of
      | adjacent buffer_op blocks.
  note: in_count counts the number of input sample per accumulation.
      |
      | The acc_end signal serves several purposes:
      |
      | 1) It captures the in_count value.
      | 2) Resets in_count for the enxt accumulation
      | 3) gets passed out to indicate that we have an accumulation's
      | worth of data  to read.
      |
      | The input samples are stored in a FIFO.  The "read_en" input pulse
      | starts a read cycle that reads out the FIFO data and drives both
      | "dout" and "valid_out".  When the read is done, the "read_next"
      | output is pulsed.  This allows several of these op_buffers to be
      | daisy chaned.
  din : Inport
  valid_in : Inport  Port=2
  acc_end : Inport  Port=3
  read_en : Inport  Port=4
  Constant : xbsIndex_r4/Constant  const=1  gui_display_data_type=Fixed-point  arith_type=Unsigned  n_bits=1  bin_pt=0  preci_type=Single  exp_width=8  frac_width=24  explicit_period=on  period=1  equ=P=C  opselect=C  inp2=PCIN>>17  opr=+  inp1=P  carry=CIN  dbl_ovrd=off  block_type=constant
  Constant1 : xbsIndex_r4/Constant  const=1  gui_display_data_type=Boolean  arith_type=Boolean  n_bits=16  bin_pt=14  preci_type=Single  exp_width=8  frac_width=24  explicit_period=on  period=1  equ=P=C  opselect=C  inp2=PCIN>>17  opr=+  inp1=P  carry=CIN  dbl_ovrd=off  block_type=constant
  Delay{2..6} (5 alike) : xbsIndex_r4/Delay  rst=off  infoeditControl=Selection of Reset will increase slice count due to use of real FFs and instead of SRLs  en=off  latency=1  dbl_ovrd=off  reg_retiming=on  block_type=delay
      settings: block_version=11.4
  FIFO : xbsIndex_r4/FIFO  mem_type=Block RAM  performance_options=First_Word_Fall_Through  infoedit1=Available only for V4, V5, V6, V7, K7, A7 and Zynq devices.  embedded_registers=on  depth=8K  percent_nbits=1  rst=off  en=off  use_dcount=off  use_percent_full_port=on  use_almost_empty=off  almost_empty_thresh=2  use_almost_full=off  almost_full_thresh=14  dbl_ovrd=off  explicit_period=off  block_type=fifo
  Logical : xbsIndex_r4/Logical  logical_function=AND  inputs=2  en=off  latency=1  precision=Full  arith_type=Unsigned  n_bits=16  bin_pt=0  align_bp=on  dbl_ovrd=off  block_type=logical
  Logical1 : xbsIndex_r4/Logical  logical_function=OR  inputs=2  en=off  latency=0  precision=Full  arith_type=Unsigned  n_bits=16  bin_pt=0  align_bp=on  dbl_ovrd=off  block_type=logical
  Relational1 : xbsIndex_r4/Relational  mode=a=b  en=off  latency=1  dbl_ovrd=off  block_type=relational
  Terminator : Terminator
  Terminator1 : Terminator
  Terminator2 : Terminator
  in_count : xbsIndex_r4/Counter  cnt_type=Free Running  cnt_to=n_buffer * n_x_op * (2 ^ (freq_chan - n_x_eng)) - 1  operation=Up  start_count=0  cnt_by_val=1  arith_type=Unsigned  n_bits=nextpow2((n_x_op/2) * (2 ^ (freq_chan - n_x_eng)))  bin_pt=0  load_pin=off  rst=on  en=on  explicit_period=off  period=1  dbl_ovrd=off  use_behavioral_HDL=off  implementation=Fabric  block_type=counter
      settings: block_version=11.4
  out_count : xbsIndex_r4/Counter  cnt_type=Free Running  cnt_to=n_buffer * n_x_op * (2 ^ (freq_chan - n_x_eng)) - 1  operation=Down  start_count=0  cnt_by_val=1  arith_type=Unsigned  n_bits=nextpow2((n_x_op/2) * (2 ^ (freq_chan - n_x_eng)))  bin_pt=0  load_pin=on  rst=off  en=on  explicit_period=off  period=1  dbl_ovrd=off  use_behavioral_HDL=off  implementation=Fabric  block_type=counter
      settings: block_version=11.4
  read_enable : xbsIndex_r4/Register  init=0  rst=on  en=on  dbl_ovrd=off  block_type=register
  dout : Outport
  valid_out : Outport  Port=2
  read_next : Outport  Port=3
  acc_end_out : Outport  Port=4
  wires:
  acc_end/1 -> Delay4/1
  in_count/1 -> out_count/2
  valid_in/1 -> Delay2/1
  read_enable/1 -> Delay6/1, Logical1/2, FIFO/3
  out_count/1 -> Relational1/2
  din/1 -> Delay3/1
  Relational1/1 -> read_next/1, [read_done]
  [read_done] -> read_enable/2
  Delay6/1 -> valid_out/1
  FIFO/1 -> Delay5/1
  Logical/1 -> acc_end_out/1
  read_en/1 -> read_enable/3
  Delay2/1 -> FIFO/2, Logical/1, in_count/2
  Delay3/1 -> FIFO/1
  Delay4/1 -> Logical/2, out_count/1, Logical1/1, in_count/1
  FIFO/2 -> Terminator/1
  FIFO/3 -> Terminator1/1
  FIFO/4 -> Terminator2/1
  Constant/1 -> Relational1/1
  Constant1/1 -> read_enable/1
  Delay5/1 -> dout/1
  Logical1/1 -> out_count/3

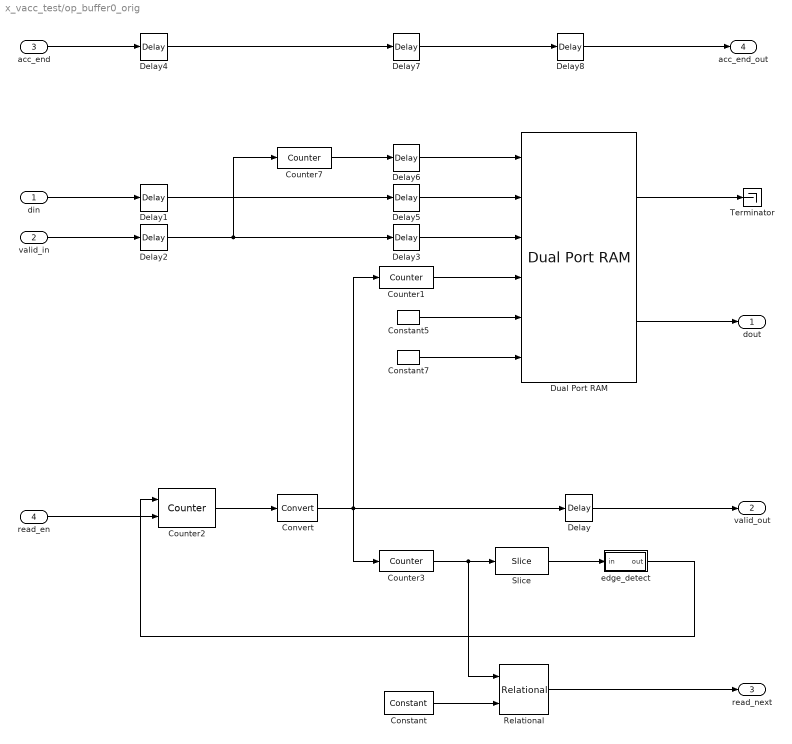
== system x_vacc_test/op_buffer0_orig ==
  din : Inport
  valid_in : Inport  Port=2
  acc_end : Inport  Port=3
  read_en : Inport  Port=4
  Constant : xbsIndex_r4/Constant  const=n_x_op * (2 ^ (freq_chan - n_x_eng)) - 2  gui_display_data_type=Fixed-point  arith_type=Unsigned  n_bits=ceil( log2( n_x_op * (2 ^ (freq_chan - n_x_eng)) - 1 ) )  bin_pt=0  preci_type=Single  exp_width=8  frac_width=24  explicit_period=on  period=1  equ=P=C  opselect=C  inp2=PCIN>>17  opr=+  inp1=P  carry=CIN  dbl_ovrd=off  block_type=constant
      settings: block_version=11.4
  Constant5 : xbsIndex_r4/Constant  const=0  gui_display_data_type=Fixed-point  arith_type=Unsigned  n_bits=64  bin_pt=0  preci_type=Single  exp_width=8  frac_width=24  explicit_period=on  period=1  equ=P=C  opselect=C  inp2=PCIN>>17  opr=+  inp1=P  carry=CIN  dbl_ovrd=off  block_type=constant
      settings: block_version=11.4
  Constant7 : xbsIndex_r4/Constant  const=0  gui_display_data_type=Fixed-point  arith_type=Boolean  n_bits=16  bin_pt=0  preci_type=Single  exp_width=8  frac_width=24  explicit_period=on  period=1  equ=P=C  opselect=C  inp2=PCIN>>17  opr=+  inp1=P  carry=CIN  dbl_ovrd=off  block_type=constant
      settings: block_version=11.4
  Convert : xbsIndex_r4/Convert  gui_display_data_type=Fixed-point  arith_type=Boolean  n_bits=16  bin_pt=14  float_type=Single  exp_bits=8  fraction_bits=24  quantization=Truncate  overflow=Wrap  en=off  latency=0  dbl_ovrd=off  pipeline=on  block_type=convert
      settings: block_version=11.4
  Counter1 : xbsIndex_r4/Counter  cnt_type=Count Limited  cnt_to=n_buffer * n_x_op * (2 ^ (freq_chan - n_x_eng)) - 1  operation=Up  start_count=0  cnt_by_val=1  arith_type=Unsigned  n_bits=ceil( log2 (n_buffer * n_x_op * (2 ^ (freq_chan - n_x_eng))))  bin_pt=0  load_pin=off  rst=off  en=on  explicit_period=off  period=1  dbl_ovrd=off  use_behavioral_HDL=off  implementation=Fabric  block_type=counter
      settings: block_version=11.4
  Counter2 : xbsIndex_r4/Counter  cnt_type=Free Running  cnt_to=1  operation=Up  start_count=0  cnt_by_val=1  arith_type=Unsigned  n_bits=1  bin_pt=0  load_pin=off  rst=on  en=on  explicit_period=off  period=1  dbl_ovrd=off  use_behavioral_HDL=off  implementation=Fabric  block_type=counter
      settings: block_version=11.4
  Counter3 : xbsIndex_r4/Counter  cnt_type=Count Limited  cnt_to=n_x_op * (2 ^ (freq_chan - n_x_eng)) - 1  operation=Up  start_count=0  cnt_by_val=1  arith_type=Unsigned  n_bits=ceil( log2 (n_x_op * (2 ^ (freq_chan - n_x_eng))))  bin_pt=0  load_pin=off  rst=off  en=on  explicit_period=off  period=1  dbl_ovrd=off  use_behavioral_HDL=off  implementation=Fabric  block_type=counter
      settings: block_version=11.4
  Counter7 : xbsIndex_r4/Counter  cnt_type=Count Limited  cnt_to=n_buffer * n_x_op * (2 ^ (freq_chan - n_x_eng)) - 1  operation=Up  start_count=0  cnt_by_val=1  arith_type=Unsigned  n_bits=ceil( log2 (n_buffer * n_x_op * (2 ^ (freq_chan - n_x_eng))))  bin_pt=0  load_pin=off  rst=off  en=on  explicit_period=off  period=1  dbl_ovrd=off  use_behavioral_HDL=off  implementation=Fabric  block_type=counter
      settings: block_version=11.4
  Delay : xbsIndex_r4/Delay  rst=off  infoeditControl=Selection of Reset will increase slice count due to use of real FFs and instead of SRLs  en=off  latency=bram_latency  dbl_ovrd=off  reg_retiming=on  block_type=delay
      settings: block_version=11.4
  Delay{1..7} (7 alike) : xbsIndex_r4/Delay  rst=off  infoeditControl=Selection of Reset will increase slice count due to use of real FFs and instead of SRLs  en=off  latency=1  dbl_ovrd=off  reg_retiming=on  block_type=delay
      settings: block_version=11.4
  Delay8 : xbsIndex_r4/Delay  rst=off  infoeditControl=Selection of Reset will increase slice count due to use of real FFs and instead of SRLs  en=off  latency=bram_latency  dbl_ovrd=off  reg_retiming=on  block_type=delay
      settings: block_version=11.4
  Dual Port RAM : xbsIndex_r4/Dual Port RAM  depth=n_buffer * n_x_op * (2 ^ (freq_chan - n_x_eng))  initVector=0  distributed_mem=Block RAM  init_a=0  init_b=0  rst_a=off  rst_b=off  en_a=off  en_b=off  latency=bram_latency  write_mode_A=Read Before Write  write_mode_B=Read Before Write  dbl_ovrd=off  optimize=Area  block_type=dpram
      settings: block_version=11.4
  Relational : xbsIndex_r4/Relational  mode=a=b  en=off  latency=1  dbl_ovrd=off  block_type=relational
  Slice : xbsIndex_r4/Slice  nbits=1  boolean_output=on  mode=Upper Bit Location + Width  bit1=0  base1=MSB of Input  bit0=0  base0=LSB of Input  dbl_ovrd=off  block_type=slice
  Terminator : Terminator
  edge_detect : Subsystem  AncestorBlock=casper_library_misc/edge_detect  mask(edge_detect){edge=Falling, polarity=Active High, x_in=[0 0.08 0.08 0.16 0.16 0.24 0.24 0.32 0.32 0.4], y_in=[1  1 -1 -1 -1 -1 -1 -1 -1 -1], x_out=[0.6 0.68 0.68 0.76 0.76 0.84 0.84 0.92 0.92 1], y_out=[-1 -1  1  1 -1 -1 -1 -1 -1 -1]}
      mask prompts: edge "Edge Type"; polarity "Output Polarity"; x_in "Input X Positions"; y_in "Input Y Positions"; x_out "Output X Positions"; y_out "Output Y Positions"
      mask help: Output is active for one-cycle after each edge of the specified type (i.e. rising, falling or both).
      mask init: same as x_vacc_test/edge_detect17
      settings: AttributesFormatString=|
      | falling edges
      | active high
      mask Help: Output is active for one-cycle after each edge of the specified type (i.e. rising, falling, or both).  Latency is zero.
      mask TypeOptions edge: Rising | Falling | Both
      mask TypeOptions polarity: Active High | Active Low
      mask Display:
      | plot(x_in_num,0.5+y_in_num*0.2);
      | plot(x_out_num,0.5+y_out_num*0.2);
  dout : Outport
  valid_out : Outport  Port=2
  read_next : Outport  Port=3
  acc_end_out : Outport  Port=4
  wires:
  Constant5/1 -> Dual Port RAM/5
  Constant7/1 -> Dual Port RAM/6
  Dual Port RAM/2 -> dout/1
  Dual Port RAM/1 -> Terminator/1
  din/1 -> Delay1/1
  valid_in/1 -> Delay2/1
  Counter7/1 -> Delay6/1
  Counter2/1 -> Convert/1
  read_en/1 -> Counter2/2
  Delay/1 -> valid_out/1
  Counter1/1 -> Dual Port RAM/4
  Counter3/1 -> Slice/1, Relational/1
  Slice/1 -> edge_detect/1(in)
  edge_detect/1(out) -> Counter2/1
  Constant/1 -> Relational/2
  Relational/1 -> read_next/1
  Convert/1 -> Delay/1, Counter1/1, Counter3/1
  Delay1/1 -> Delay5/1
  Delay2/1 -> Counter7/1, Delay3/1
  acc_end/1 -> Delay4/1
  Delay4/1 -> Delay7/1
  Delay3/1 -> Dual Port RAM/3
  Delay5/1 -> Dual Port RAM/2
  Delay6/1 -> Dual Port RAM/1
  Delay7/1 -> Delay8/1
  Delay8/1 -> acc_end_out/1
